simulink model "ofdm_txrx_supermimo_coded"  (Simulink 7.1)
settings: Solver=VariableStepDiscrete  SolverMode=SingleTasking  StartTime=0.0  StopTime=2*simOnly_simLength  RelTol=1e-3
PostLoadFcn:
  | ofdm_rx_supermimo_init
InitFcn:
  | ofdm_rx_supermimo_init
StartFcn:
  | ofdm_rx_supermimo_init
values from neighbouring files (symbols this model uses):
  Action0_Config = 0* 31*2^18 + 0*2^24 + PHY_AUTORESPONSE_REQ_GOODHDR + PHY_AUTORESPONSE_REQ_GOODPKT + PHY_AUTORESPONSE_REQ_FLAGA    [ofdm_tx_supermimo_init.m]
  Action1_Config = 0* 62*2^18 + PHY_AUTORESPONSE_REQ_GOODHDR + PHY_AUTORESPONSE_REQ_GOODPKT    [ofdm_tx_supermimo_init.m]
  Action2_Config = 0    [ofdm_tx_supermimo_init.m]
  Action3_Config = 0    [ofdm_tx_supermimo_init.m]
  Action4_Config = 0    [ofdm_tx_supermimo_init.m]
  Action5_Config = 0    [ofdm_tx_supermimo_init.m]
  ActionID_AFTransmit = 31    [ofdm_tx_supermimo_init.m]
  ActionID_Disabled = 0    [ofdm_tx_supermimo_init.m]
  ActionID_SetFlagA = 62    [ofdm_tx_supermimo_init.m]
  ActionID_SetFlagB = 61    [ofdm_tx_supermimo_init.m]
  BER_RAM_init_size = 2048    [ofdm_tx_supermimo_init.m]
  channel_h = [1 0; 1 0] * 0.3    [ofdm_tx_supermimo_init.m]
  CPLength = 16    [ofdm_tx_supermimo_init.m]
  FEC_Config = fec_coding_en*1 + fec_soft_dec*2 + fec_qpsk_scl*2^4 + fec_16qam_scl*2^8    (= 4195)    [ofdm_tx_supermimo_init.m]
  longCorr_coef_i = [-4*real(LTS) 0]    [ofdm_rx_supermimo_init.m]
  longCorr_coef_q = [4*imag(LTS) 0]    [ofdm_rx_supermimo_init.m]
  Match0_Config = 0    [ofdm_tx_supermimo_init.m]
  Match1_Config = 0    [ofdm_tx_supermimo_init.m]
  Match2_Config = 0    [ofdm_tx_supermimo_init.m]
  Match3_Config = 0    [ofdm_tx_supermimo_init.m]
  Match4_Config = 0    [ofdm_tx_supermimo_init.m]
  Match5_Config = 0    [ofdm_tx_supermimo_init.m]
  Match6_Config = 0    [ofdm_tx_supermimo_init.m]
  Match7_Config = 0    [ofdm_tx_supermimo_init.m]
  max_CP_length = 16    [ofdm_tx_supermimo_init.m]
  max_num_baseRateSymbols = 31    [ofdm_tx_supermimo_init.m]
  max_num_subcarriers = 64    [ofdm_tx_supermimo_init.m]
  max_num_trainingSymbols = 15    [ofdm_tx_supermimo_init.m]
  max_numBytes = 16384    [ofdm_tx_supermimo_init.m]
  max_OFDM_symbols = 1023    [ofdm_tx_supermimo_init.m]
  modConstellation_qam16 = .75*[1 3 -1 -3]./3    [ofdm_tx_supermimo_init.m]
  modConstellation_qam64 = 0.875*[3 1 5 7 -3 -1 -5 -7]./7    [ofdm_tx_supermimo_init.m]
  modConstellation_qpsk = [1 -1]./sqrt(2)    [ofdm_tx_supermimo_init.m]
  numBaseRateSymbols = numBaseRateSymbols *2    [ofdm_tx_supermimo_init.m]
  numSubcarriers = 64    [ofdm_tx_supermimo_init.m]
  numTrainingSymbols = 2    [ofdm_tx_supermimo_init.m]
  pilot_indicies = 7 + ( (64-21) * 2^8) + ( (64-7) * 2^16) + (21 * 2^24)    (= 356068103)    [ofdm_tx_supermimo_init.m]
  pilotValues = hex2dec('A57D') + (2^16 * hex2dec('5A82'))    [ofdm_tx_supermimo_init.m]
  pktByteNums = numHeaderBytes +  				byteIndex_numPayloadBytes(1)*2^8 +  				byteIndex_numPayloadBytes(2)*2^16 +  				byteIndex_simpleDynModMasks * 2^24    [ofdm_rx_supermimo_init.m]
  PktDet_Delay = 58+32 +      Symbol_Timing_Offset*2^7 +      0*2^16 +      (117* 2^24)    (= 1962935642)    [ofdm_rx_supermimo_init.m]
  pktTiming_count_payloadStart = pktTiming_count_lsStart + pktTiming_ls_num*pktTiming_ls_length    [ofdm_rx_supermimo_init.m]
  preamble = preamble_scale*[shortsyms_10 longsyms_2]    [ofdm_tx_supermimo_init.m]
  preamble_ROM = preamble_I_ROM + preamble_Q_ROM*2^16    [ofdm_tx_supermimo_init.m]
  RAM_init_size = max_numBytes    (= 16384)    [ofdm_tx_supermimo_init.m]
  reg_AF_Tx_Blanking = (320+80) + ( (320+80+79)*2^16)    (= 31392144)    [ofdm_rx_supermimo_init.m]
  reg_AF_Tx_Scaling = 2944    [ofdm_rx_supermimo_init.m]
  reg_Interrupt_PktBuf_Control = reg_InterruptControl +  	1 * 2^16 +  	0 * 2^24    (= 65536)    [ofdm_rx_supermimo_init.m]
  reg_PilotCalcParams = (4 * 2^16) + (minPilotChanMag)    (= 262184.96)    [ofdm_rx_supermimo_init.m]
  regRx_chanEst_minMag = ((0.5)*2^15) + ((0.5 * 2^15)*2^16)    (= 1073758208)    [ofdm_rx_supermimo_init.m]
  regRx_coarseCalcCorrection = round((1.3e-3 - 1.2982e-3)*2^32)    [ofdm_rx_supermimo_init.m]
  regRx_pilotCalcCorrection = round(1.00115 * 2^31)    [ofdm_rx_supermimo_init.m]
  regRx_preCFOoptions = 0 * 2^0 +  	1 * 2^1 +  	0    (= 2)    [ofdm_rx_supermimo_init.m]
  rx_controlBits = 1 * 2  		 + 1 * 4  		 + 0 * 8  		 + 1 * 16 * rx_SISO_Mode  		 + 1 * 32  		 + 1 * 64  		 + 1 * 128  		 + 1 * 256  		 + 1 * 512  		 + 1 * 1024  		 + 1 * 4096  		 + 0 * 16384  		 + 0 * 32768  		 + 1 * 65536  		 + 0 * 131072  		 + 0 * 262144  		 + 1 * 524288  		 + 1 * 1048576 * tx_Alamouti_Mode  		 + 0 * 2097152           + 1 * 33554432  		 + 0 * 4194304  		 + 0 * 8388608  		 + 0 * 16777216  		 + 1 *  ...(+49 chars)    [ofdm_rx_supermimo_init.m]
  Rx_PktDet_LongCorr_Params = LongCorrCounterLoad + 0*2^8 + 0*2^16 + 255*2^24    (= 4278190325)    [ofdm_rx_supermimo_init.m]
  Rx_PktDet_LongCorrThresholds = 8000    [ofdm_rx_supermimo_init.m]
  rx_postEq_scaling = round(rxScaling*2^11) * (1 + 2^16)    [ofdm_rx_supermimo_init.m]
  RxDataScrambling_Seq = [40 198 78 63 82 173 102 245 48 111 172 115 147 230 216 93 72 65 62 2 205 242 122 90 128 83 105 97 73 10 5 252]    [ofdm_tx_supermimo_init.m]
  RxReg_FixedPktLen = 0    [ofdm_rx_supermimo_init.m]
  rxReg_pktDetCorr = 2^00 * (floor(0.7*128)) +  	2^08 * (300) +  	0    [ofdm_rx_supermimo_init.m]
  shortSymbol_time = shortSymbol_time(1:16).'    [ofdm_tx_supermimo_init.m]
  simOnly_numSamples = length(preamble)+( (numSubcarriers+CPLength)*(numTrainingSymbols + numBaseRateSymbols + numFullRateSymbols/2) )    [ofdm_tx_supermimo_init.m]
  train = [train train]    [ofdm_tx_supermimo_init.m]
  tx_controlBits = (antB_preambleShift * 2^4) + (2^1 * tx_Alamouti_Mode) +  	(2^0 * tx_SISO_Mode) + 4*0 + 8*1 + 0*256 + 512*0 + 1024*0 +  	0*hex2dec('1000') + 0*hex2dec('2000') + 0*hex2dec('4000') +  	1*hex2dec('8000') + 1*hex2dec('10000') + hex2dec('20000') +  	0*hex2dec('100000')    [ofdm_tx_supermimo_init.m]
  tx_delays = (50*2^0) + (0*2^8) + (30*2^12)    (= 122930)    [ofdm_tx_supermimo_init.m]
  tx_scaling = (3072) + (12288 * 2^16)    (= 805309440)    [ofdm_tx_supermimo_init.m]
  TxDataScrambling_Seq = [40 198 78 63 82 173 102 245 48 111 172 115 147 230 216 93 72 65 62 2 205 242 122 90 128 83 105 97 73 10 5 252]    [ofdm_tx_supermimo_init.m]
  txReg_symbolCounts = (2^8 *numBaseRateSymbols ) + numTrainingSymbols    [ofdm_tx_supermimo_init.m]
  TxRx_FFTScaling = (3 * 2^0) +  	(2 * 2^2) +  	(1 * 2^4) +  	(1 * 2^6) +  	(1 * 2^8) +  	(0 * 2^10)    (= 347)    [ofdm_tx_supermimo_init.m]
undefined symbols (not defined in the file or in its folder): AntA, AntB, BaseRate, Down, gcbh, numBuffs, numBytes, Up, xlBlockLoadCallback, xlGetSimulinkPeriod, xlipgetpartsetting
libraries: xbsIndex_r4 x6000, simulink x4, dspsigops x4, commchan3 x2, dspsrcs4 x2, commrflib2 x2
(part 3 of 63)

== system ofdm_txrx_supermimo_coded/Memory-mapped Registers ==
  AutoReply Registers : Subsystem
  Rx Registers : Subsystem
  Static Parameters (might be registers someday) : Subsystem
  Tx Registers : Subsystem
  TxRx Registers : Subsystem

== system ofdm_txrx_supermimo_coded/OFDM Rx MIMO ==
  AntA_I : Inport  OutDataType=sfix(16)  OutScaling=2^0
  AntA_Q : Inport  Port=2  OutDataType=sfix(16)  OutScaling=2^0
  AntB_I : Inport  Port=3  OutDataType=sfix(16)  OutScaling=2^0
  AntB_Q : Inport  Port=4  OutDataType=sfix(16)  OutScaling=2^0
  ADC Inputs & Antenna Selection : Subsystem
  AF Buffers : Subsystem
  ChipScope : Subsystem
  Coarse Freq Correction : Subsystem
  Display : Display
  Display1 : Display
  Display2 : Display
  Divide : Product  Inputs=*/  OutDataType=sfix(16)  OutScaling=2^-10
  Equalizer & Packetizer : Subsystem
  FFT & Chan Est : Subsystem
  Long Corr HP : Subsystem
  Packet_Detection : Subsystem
  Packet_Timing_Control : Subsystem
  Rx Input : Scope  NumInputPorts=7
      settings: TimeRange=14600  YMin=-0.2~-0.2~-1~-1~0~0~0  YMax=0.2~0.2~1~1~1~22500~1  SaveName=ScopeData48
  Rx Reset Logic : Subsystem
  done{1..9} (9 alike) : xbsIndex_r4/Gateway Out  hdl_port=off  timing_constraint=None  locs_specified=off  LOCs={}  block_type=gatewayout
      settings: infoedit="Gateway out block.  Converts Xilinx fixed point inputs into ouputs of type Simulink integer, double, or fixed point.<P><P>Hardware notes:  In hardware these blocks become top level output ports or are discarded, depending on how they are configured."  xl_area=[0 0 0 0 1 0 0]  block_version=VER_STRING_GOES_HERE
  tag scope: Payload=global, PktDetected=global
  wires:
  Packet_Detection/1(Pkt Det) -> Packet_Timing_Control/1(Pkt Det), done1/1
  Packet_Timing_Control/1(Payload_Preamble) -> Equalizer & Packetizer/1(Payload), FFT & Chan Est/1(Payload), Packet_Detection/3(Payload), AF Buffers/2(Payload), [Payload], done7/1
  Long Corr HP/1(y) -> Packet_Timing_Control/2(Long CorrA), done2/1
  done1/1 -> Rx Input/5
  done4/1 -> Rx Input/1
  done5/1 -> Rx Input/2
  done3/1 -> Rx Input/3
  done6/1 -> Rx Input/4
  Coarse Freq Correction/2("AntA Q ") -> FFT & Chan Est/3(AntA Q), Long Corr HP/2(Q In)
  Coarse Freq Correction/3("AntB I ") -> FFT & Chan Est/4(AntB I)
  Coarse Freq Correction/4("AntB Q ") -> FFT & Chan Est/5(AntB Q)
  done2/1 -> Rx Input/6
  AntA_I/1 -> ADC Inputs & Antenna Selection/2("A I ")
  AntA_Q/1 -> ADC Inputs & Antenna Selection/3("A Q ")
  AntB_I/1 -> ADC Inputs & Antenna Selection/4("B I ")
  AntB_Q/1 -> ADC Inputs & Antenna Selection/5("B Q ")
  Equalizer & Packetizer/1(done) -> Packet_Timing_Control/3(Done), AF Buffers/1(PktDone)
  FFT & Chan Est/1(Y_I) -> Equalizer & Packetizer/2(Y_I)
  FFT & Chan Est/2(Y_Q) -> Equalizer & Packetizer/3(Y_Q)
  FFT & Chan Est/3(H-I) -> Equalizer & Packetizer/4(H-I)
  FFT & Chan Est/4(H-Q) -> Equalizer & Packetizer/5(H-Q)
  FFT & Chan Est/5(SubCar Index) -> Equalizer & Packetizer/6(SubCar Index)
  FFT & Chan Est/6(Sym Index) -> Equalizer & Packetizer/7(Sym Index)
  FFT & Chan Est/7(Vout) -> Equalizer & Packetizer/8(Vin)
  Packet_Timing_Control/2(PktReset) -> ADC Inputs & Antenna Selection/1(PktDetReset), Coarse Freq Correction/1(Reset), Packet_Detection/2(Reset)
  Packet_Timing_Control/3(PktDetected) -> [PktDetected], Coarse Freq Correction/2(PktDet), Packet_Detection/1(Pkt Active)
  Divide/1 -> Display/1
  [regRx_BER_Errors] -> done8/1
  [regRx_BER_TotalBits] -> done9/1
  ADC Inputs & Antenna Selection/1(A I) -> Coarse Freq Correction/3(AntA I), done4/1, AF Buffers/3(AntA_I)
  ADC Inputs & Antenna Selection/2(A Q) -> Coarse Freq Correction/4(AntA Q), done5/1, AF Buffers/4(AntA_Q)
  ADC Inputs & Antenna Selection/3(B I) -> Coarse Freq Correction/5(AntB I), done3/1
  ADC Inputs & Antenna Selection/4(B Q) -> Coarse Freq Correction/6(AntB Q), done6/1
  FFT & Chan Est/8(Phase I) -> Equalizer & Packetizer/9(Phases I)
  Coarse Freq Correction/1("AntA I ") -> FFT & Chan Est/2(AntA I), Long Corr HP/1(I In)
  done7/1 -> Rx Input/7
  FFT & Chan Est/9(Phase Q) -> Equalizer & Packetizer/10(Phases Q)
  done8/1 -> Divide/1, Display1/1
  done9/1 -> Divide/2, Display2/1

== system ofdm_txrx_supermimo_coded/OFDM Tx MIMO ==
  IFFT : Subsystem
  OutputBuffers : Subsystem
  OutputMuxes : Subsystem
  "Pre-Spin,  Filters & DACs" : Subsystem
  Training_Data : Subsystem  Orientation=left
  Tx Reset Logic : Subsystem
  TxControl : Subsystem
  TxStart : Subsystem
  AntA I : Outport  OutDataType=sfix(16)  OutScaling=2^0
  AntA Q : Outport  Port=2  OutDataType=sfix(16)  OutScaling=2^0
  AntB I : Outport  Port=3  OutDataType=sfix(16)  OutScaling=2^0
  AntB Q : Outport  Port=4  OutDataType=sfix(16)  OutScaling=2^0
  wires:
  TxStart/1(Start) -> TxControl/1(StartTx)
  Training_Data/1(tx_Q) -> IFFT/3(xn_Q)
  TxControl/7(Start IFFT) -> IFFT/4(Start)
  IFFT/3(xk_I) -> OutputBuffers/2(I)
  IFFT/4(xk_Q) -> OutputBuffers/3(Q)
  IFFT/1(xn_Index) -> Training_Data/7(xn_index)
  IFFT/5(xk_Index) -> OutputBuffers/4(Xk_index)
  OutputBuffers/1(AntA_I) -> OutputMuxes/2(AntA I)
  OutputBuffers/2(AntA_Q) -> OutputMuxes/3(AntA Q)
  TxControl/5(Training_Data) -> Training_Data/2(train_pay)
  TxControl/6(CPn_Data) -> Training_Data/1(CPn_Data)
  TxControl/4(BaseRate_En) -> Training_Data/3(BaseRate_En)
  OutputBuffers/5(Vout) -> OutputMuxes/6(vin)
  Training_Data/2(tx_I) -> IFFT/2(xn_I)
  OutputBuffers/6(Pkt Done) -> OutputMuxes/7(Pkt Done), Training_Data/6(packet_done), IFFT/5(Pkt Done), TxControl/2(PktDone)
  OutputBuffers/3(AntB_I) -> OutputMuxes/4(AntB I)
  OutputBuffers/4(AntB_Q) -> OutputMuxes/5(AntB Q)
  TxControl/1(Preamble Index) -> OutputMuxes/1(Pream Index)
  IFFT/2(Vout) -> OutputBuffers/1(IFFT Vout)
  TxControl/3(FFT En) -> Training_Data/4(FFT En)
  "Pre-Spin,  Filters & DACs"/1(" A_I") -> AntA I/1
  "Pre-Spin,  Filters & DACs"/2(" A_Q") -> AntA Q/1
  "Pre-Spin,  Filters & DACs"/3(" B_I") -> AntB I/1
  "Pre-Spin,  Filters & DACs"/4(" B_Q") -> AntB Q/1
  TxControl/2(Start) -> Training_Data/5(Start)
  Training_Data/3(Payload Done) -> IFFT/1(Payload Done)
  IFFT/6(LastSym) -> OutputBuffers/5(Last Sym)
  OutputMuxes/1(Payload) -> "Pre-Spin,  Filters & DACs"/1(Payload)
  OutputMuxes/2(" AntA I") -> "Pre-Spin,  Filters & DACs"/2(A_I)
  OutputMuxes/3(" AntA Q") -> "Pre-Spin,  Filters & DACs"/3(A_Q)
  OutputMuxes/4(" AntB I") -> "Pre-Spin,  Filters & DACs"/4(B_I)
  OutputMuxes/5(" AntB Q") -> "Pre-Spin,  Filters & DACs"/5(B_Q)

== system ofdm_txrx_supermimo_coded/Pkt Buffer BRAM Interface ==
  Addr Select : Subsystem
  BRAM_Addr : xbsIndex_r4/Gateway Out  hdl_port=on  timing_constraint=None  locs_specified=off  LOCs={}  block_type=gatewayout
      settings: infoedit="Gateway out block.  Converts Xilinx fixed point inputs into ouputs of type Simulink integer, double, or fixed point.<P><P>Hardware notes:  In hardware these blocks become top level output ports or are discarded, depending on how they are configured."  xl_area=[0, 0, 0, 0, 0, 0, 0]  block_version=VER_STRING_GOES_HERE
  BRAM_DataIn : xbsIndex_r4/Gateway In  arith_type=Unsigned  n_bits=64  bin_pt=0  quantization="Round  (unbiased: +/- Inf)"  overflow=Saturate  period=1  dbl_ovrd=off  timing_constraint=None  locs_specified=off  LOCs={}  block_type=gatewayin
      settings: infoedit="Gateway in block.  Converts inputs of type Simulink integer, double and fixed point to  Xilinx fixed point type.<P><P>Hardware notes:  In hardware these blocks become top level input ports."  block_version=VER_STRING_GOES_HERE
  BRAM_Dout : xbsIndex_r4/Gateway Out  hdl_port=on  timing_constraint=None  locs_specified=off  LOCs={}  block_type=gatewayout
      settings: infoedit="Gateway out block.  Converts Xilinx fixed point inputs into ouputs of type Simulink integer, double, or fixed point.<P><P>Hardware notes:  In hardware these blocks become top level output ports or are discarded, depending on how they are configured."  xl_area=[0, 0, 0, 0, 0, 0, 0]  block_version=VER_STRING_GOES_HERE
  BRAM_Reset : xbsIndex_r4/Gateway Out  hdl_port=on  timing_constraint=None  locs_specified=off  LOCs={}  block_type=gatewayout
      settings: infoedit="Gateway out block.  Converts Xilinx fixed point inputs into ouputs of type Simulink integer, double, or fixed point.<P><P>Hardware notes:  In hardware these blocks become top level output ports or are discarded, depending on how they are configured."  block_version=8.2.02
  BRAM_WEn : xbsIndex_r4/Gateway Out  hdl_port=on  timing_constraint=None  locs_specified=off  LOCs={}  block_type=gatewayout
      settings: infoedit="Gateway out block.  Converts Xilinx fixed point inputs into ouputs of type Simulink integer, double, or fixed point.<P><P>Hardware notes:  In hardware these blocks become top level output ports or are discarded, depending on how they are configured."  xl_area=[0, 0, 0, 0, 0, 0, 0]  block_version=VER_STRING_GOES_HERE
  Constant : xbsIndex_r4/Constant  arith_type=Unsigned  const=0  n_bits=1  bin_pt=0  explicit_period=on  period=1  equ=P=C  opselect=C  inp2=PCIN>>17  opr=+  inp1=P  carry=CIN  dbl_ovrd=off  block_type=constant
      settings: block_version=8.2.02
  Mux : xbsIndex_r4/Mux  inputs=2  en=off  latency=0  precision=Full  arith_type=Unsigned  n_bits=16  bin_pt=14  quantization=Truncate  overflow=Wrap  dbl_ovrd=off  block_type=mux
      settings: block_version=8.2.02
  PLB Pkt Buffer : Subsystem
  Pkt Buffer Addressing : Subsystem
  SIM ONLY! FlexBER Pkt Buffer : Subsystem
  tag scope: BRAM_Tx_DIn=global, Debug_BRAM_Addr=global
  wires:
  PLB Pkt Buffer/1(Data) -> BRAM_DataIn/1
  BRAM_DataIn/1 -> [BRAM_Tx_DIn]
  Constant/1 -> BRAM_Reset/1
  Mux/1 -> BRAM_Addr/1, [Debug_BRAM_Addr]
  BRAM_Dout/1 -> PLB Pkt Buffer/1(BRAM_Dout)
  BRAM_WEn/1 -> PLB Pkt Buffer/2(WEn)
  BRAM_Addr/1 -> PLB Pkt Buffer/3(Addr)
  Pkt Buffer Addressing/1(Tx_RdAddr) -> Mux/2
  Pkt Buffer Addressing/2(Rx_WrAddr) -> Mux/3
  Addr Select/1(Sel) -> Mux/1
  [BRAM_Rx_WrEn] -> BRAM_WEn/1
  [BRAM_Rx_DOut] -> BRAM_Dout/1

== system ofdm_txrx_supermimo_coded/Workspace Transmitter ==
  From Workspace : FromWorkspace  VariableName=rxAntI  SampleTime=4  Interpolate=off  OutputAfterFinalValue=Cyclic repetition
  From Workspace1 : FromWorkspace  VariableName=rxAntQ  SampleTime=4  Interpolate=off  OutputAfterFinalValue=Cyclic repetition
  RXreset : Constant  Value=0  OutDataType=sfix(16)  OutScaling=2^0  SampleTime=4
  A_I : Outport  OutDataType=sfix(16)  OutScaling=2^0
  A_Q : Outport  Port=2  OutDataType=sfix(16)  OutScaling=2^0
  B_I : Outport  Port=3  OutDataType=sfix(16)  OutScaling=2^0
  B_Q : Outport  Port=4  OutDataType=sfix(16)  OutScaling=2^0
  wires:
  From Workspace/1 -> A_I/1
  From Workspace1/1 -> A_Q/1
  RXreset/1 -> B_I/1, B_Q/1

== system ofdm_txrx_supermimo_coded/"Channel"/Delays ==
  In1 : Inport  OutDataType=sfix(16)  OutScaling=2^0
  In2 : Inport  Port=2  OutDataType=sfix(16)  OutScaling=2^0
  Integer Delay1 : simulink/Discrete/Integer Delay  vinit=0.0  samptime=-1  NumDelays=4
  Integer Delay2 : simulink/Discrete/Integer Delay  vinit=0.0  samptime=-1  NumDelays=4
  Out1 : Outport  OutDataType=sfix(16)  OutScaling=2^0
  Out2 : Outport  Port=2  OutDataType=sfix(16)  OutScaling=2^0
  wires:
  In1/1 -> Integer Delay1/1
  Integer Delay1/1 -> Out1/1
  Integer Delay2/1 -> Out2/1
  In2/1 -> Integer Delay2/1

== system ofdm_txrx_supermimo_coded/"Channel"/Freq Offset ==
  note: Optional additive carrier
      | Models effects of DC-offsets at Tx/Rx
      | Set gain=0.15 for realistic offset
  In1 : Inport  OutDataType=sfix(16)  OutScaling=2^0
  In2 : Inport  Port=2  OutDataType=sfix(16)  OutScaling=2^0
  Product4 : Product  OutDataType=sfix(16)  OutScaling=2^0  RndMeth=Zero
  Product5 : Product  OutDataType=sfix(16)  OutScaling=2^0  RndMeth=Zero
  Sine Wave : dspsrcs4/Sine Wave  Amplitude=1  Frequency=freqOffsetB/4  Phase=0  SampleMode=Discrete  OutComplex=Complex  CompMethod=Trigonometric fcn  TableSize=Speed  SampleTime=1  SamplesPerFrame=1  dataType=double  wordLen=16  udDataType=sfix(16)  fracBitsMode=Best precision  numFracBits=15  ResetState=Restart at time zero
  Sine Wave1 : dspsrcs4/Sine Wave  Amplitude=1  Frequency=freqOffsetA/4  Phase=0  SampleMode=Discrete  OutComplex=Complex  CompMethod=Trigonometric fcn  TableSize=Speed  SampleTime=1  SamplesPerFrame=1  dataType=double  wordLen=16  udDataType=sfix(16)  fracBitsMode=Best precision  numFracBits=15  ResetState=Restart at time zero
  Out1 : Outport  OutDataType=sfix(16)  OutScaling=2^0
  Out2 : Outport  Port=2  OutDataType=sfix(16)  OutScaling=2^0
  tag scope: freqOffset=global
  wires:
  Sine Wave/1 -> Product5/2
  In1/1 -> Product4/1
  In2/1 -> Product5/1
  Sine Wave1/1 -> Product4/2, [freqOffset]
  Product4/1 -> Out1/1
  Product5/1 -> Out2/1

== system ofdm_txrx_supermimo_coded/"Channel"/MIMO "Channel" ==
  In1 : Inport  OutDataType=sfix(16)  OutScaling=2^0
  In2 : Inport  Port=2  OutDataType=sfix(16)  OutScaling=2^0
  Add : Sum  OutDataType=sfix(16)  OutScaling=2^-10
  Add1 : Sum  OutDataType=sfix(16)  OutScaling=2^-10
  Constant : Constant  Value=h(1,1)  OutDataType=sfix(16)  OutScaling=2^0
  Constant1 : Constant  Value=h(2,1)  OutDataType=sfix(16)  OutScaling=2^0
  Constant2 : Constant  Value=h(1,2)  OutDataType=sfix(16)  OutScaling=2^0
  Constant3 : Constant  Value=h(2,2)  OutDataType=sfix(16)  OutScaling=2^0
  Display{,1..3} (4 alike) : Display
  Product{,1..3} (4 alike) : Product  OutDataType=sfix(16)  OutScaling=2^0  RndMeth=Zero
  Out1 : Outport  OutDataType=sfix(16)  OutScaling=2^0
  Out2 : Outport  Port=2  OutDataType=sfix(16)  OutScaling=2^0
  wires:
  Constant/1 -> Product/2, Display/1
  Product/1 -> Add/1
  In1/1 -> Product/1, Product2/1
  In2/1 -> Product1/2, Product3/2
  Constant1/1 -> Product1/1, Display1/1
  Product1/1 -> Add/2
  Constant2/1 -> Product2/2, Display2/1
  Product2/1 -> Add1/1
  Constant3/1 -> Product3/1, Display3/1
  Product3/1 -> Add1/2
  Add/1 -> Out1/1
  Add1/1 -> Out2/1

== system ofdm_txrx_supermimo_coded/Changelog/Through PHY 3.00i ==
  note: Export 2.01.r:
      | -Yet another pilot phase fix
  note: Export 2.01.s:
      | -Disabled the CFO correction filter (to save multipliers in the V4)
  note: Export 2.01.t:
      | -Changed SISO H_BB matrix value to 1 (from 0.5+0.5j)
  note: ========================================
      | Starting iterations for pre-spin/DF support
  note: Export 3.00.a:
      | -Started from 2.01.t (PHY for refdes14.1_fpgaV2_public)
      | -Re-generated memmap (sims are faster w/out EDK Processor block)
      | -Removed nearly all scopes (faster sims, less memory consumption)
      | -Removed match6/7 blocks and localHeader buffer in autoResponse system (we never used this,
      |   after figuring the flagA/flagB actions/conditions were enough)
      | -Removed CFO PI filter
      | -Moved phase normalization to immediately after pilot arctan
      | -Completely redesigned pilot insertion logic and phase estimation/tracking logic. Pilots in Alamouti mode
      |    are now interleaved in frequency, not time. Phase error corrections are applied to the current symbol,
      |    not the next one. Overall improvement in EVM should be good, especially in Alamouti mode.
      | -Fixed checksum bug in random payload mode (payloads were being flagged as all bad)
      | -Added readback registers for pilot-based and coarse CFO estimates
      | -Added readback register for whether A and B channels are used in pilot phase calculation (Alamouti mode only)
  note: Export 3.00.b:
      | -Added FFT overflow/AGC/pkt signals to ChipScope
  note: Export 3.00.c:
      | -Fixed pilot selection for single-path Alamouti
  note: Export 3.00.d:
      | -Added CFO pre-spin blocks
      |   -Multipliers in Tx path, before filters
      |   -Pilot-based CFO estimator
      |   -Per-packet-buffer CFO capture buffer
  note: Export 3.00.e:
      | -Changed pre-spin mults to conj-mults
  note: Export 3.00.f:
      | -Added pktDetection event counter
      | -Added logic to capture random Tx payloads to a pkt buffer
      | -Shifted autoTx delay up one bit (user's 8 bits are [8:1] of 9-bit delay)
      | -Fixed antB conj mult (was conjugating wrong argument)
  note: Export 3.00.g:
      | -Fixed autoTx delay comparison (to < instead of <=, so that Tx isn't always being asserted)
  note: Export 3.00.h:
      | -Fixed latency on pilotCFOest_en signals, bumped CFO capture buffer to T=1
      | -Fixed autoTx delay counter (now 9 bits, matching delay param)
      | -Updated LFSR polynomial
  note: Export 3.00.i:
      | -Redesigned pilot pre-CFO calculator, to use all received phases instead of trying to average just the last N.

== system ofdm_txrx_supermimo_coded/Changelog/Through PHY 3.00u ==
  note: Export 3.00.j:
      | -Fixed bug in pilot-CFO calc (added reset to unwrapping counter)
  note: Export 3.00.k:
      | -Fixed bug in random payload generation/recording (LFSR en toggled only once per pkt in 3.00j)
  note: Export 3.00.l:
      | -Fixed random payload mode (again) so capture & checksums will work.
  note: Export 3.00.m:
      | -Added precision and multiplication correction factor to pilotCFO estimator, based on experimental tests.
  note: Export 3.00.n:
      | -Added AutoTwoTx circuit, to optionally transmit every user-initiated packet twice with programmed delay
      | -Added external PktDetReset input to hold Rx PHY in reset via GPIO
  note: Export 3.00.o:
      | -Fixed timing of AutoTwoTx
      | -Added autoResponse action to force Tx antenna swap
      | -Added optional circuit to calculate/store channel magnitudes (and not raw I/Q)
  note: Export 3.00.p:
      | -Added hooks to disable random payload generation for autoResponse and autoTwoTx transmissions
      |    (don't want new payloads for slot2 when testing PHY stuff)
      | -Fixed pktBuf mixup on autoTwoTx transissions (shouldn't use autoResponse pktBuf assignment)
  note: Export 3.00.q:
      | -Rearranged coarse CFO calc (moved 1/2pi scaling just after arctan)
      | -Added coarseCFO correction adder
  note: Export 3.00.r:
      | -Added coarseCFO correction register to EDK processor block (was omitted in q)
  note: Export 3.00.t:
      | -Redesigned checksum subsystems; header now has real CRC-16, full packet has real CRC-32
  note: Export 3.00.s:
      | -Re-export of 3.00r with logic multiliers for V4 project
  note: Export 3.00.u:
      | -Added EVM buffers
      |   -One summing all EVM for a given subcarrier across symbols
      |   -One summing EVM across all subcarriers per symbol
      | -Changed antB preamble shift to add (delay relative to antA)
      | -Changed autoResponder delay to 9 bits {user8, 0}, so each user bit is a sample delay
      | -Renamed coarseCFO correction register

== system ofdm_txrx_supermimo_coded/Changelog/Through PHY 3.01f ==
  note: Export 3.00.v:
      | -Fixed EVM-per-sc logic to reset at start of each Rx packet
  note: Export 3.00.w:
      | -Fixed autoResponder timing (new CRC blocks were 2 cycles slower declaring good/bad payload)
  note: Export 3.00.x:
      | -Added extra delay bits for autoTx and reTx counters
      | -Increased int. bits for per-SC EVM accumulation
      | -Added external pktDet port
      | -Rearragned TxControlBits register bits
  note: Export 3.00.y:
      | -Added additional TxRunning outputs with programmable masks/delays (for faking pktDetecton)
      | -Rearranged TxControlBits register and added TxDelays register
  note: Export 3.00.z:
      | -Added logic to allow coarse CFO to be triggered only by external pktDet (so long correlation can be bypassed entirely)
      | -Added channel estimate magnitude checking to optionally ignore HAA or HBA when they're too small
      | -Added logic to optionally mask part of the AF transmission (to blank the now-noisy unused training symbol)
      | -Changed AF scaling so all AF samples are scaled by TxPayload scaling (only re-generated preamble gets scaled
      |     by TxPreamble scaling)
  note: Export 3.01.a:
      | -Fixed timing bug that blocked autoResponder flags
  note: Export 3.01.b:
      | -Added selectable filters for TxA I/Q
  note: Export 3.01.c:
      | -Added precision to long correlator
  note: Export 3.01.d:
      | -Added registers to mask input I/Q until AGC is done
  note: Export 3.01.e:
      | -Added extra longCorr threshold check
  note: Export 3.01.f:
      | -Removed dualThreshold logic (didn't help)
      | -Rebuilt preamble generator for realy cyclic shift of STS/LTS for antB (removed masking too)
      | -Added regs to fix length of Rx pkt (for testing "true" AF)
      | -Removed unused RxControlBits (reqShortCorr, dynPktLenEn)
      | -Added windowing for LongCorr
      | -Added optional conjugation of antB preamble
      | -Added conjugate-correlation output from LongCorr

== system ofdm_txrx_supermimo_coded/Changelog/Through refdes14.0 ==
  note: Export 2.00.x:
      | -Added register to capture autoTx delay (required for actions which require a Flag)
      | -Added better TxStart logic (posedge on TxStart gateway; OR'd AF_TxDone for TxRunning SR reset)
      | -Screwed with precision in equalizer (more fractional bits, knowing abs(H)<1)
      | -Register phase error to chipscope/debug port
      | -Fixed TxPktLength%12==0 problem
      | -Adjust pktDet RSSI for RF gain change
      | -Added muxes to bypass Tx interp filters
  note: Export 2.00.y:
      | -Added option to use diff(phaseError) for fine CFO
      | -Shortened coarse CFO window to 68 samles (from 72)
      | -CFO delay should be > 0 (already settable in software)
      | -Increased precision of input to phase error scaling/lookup (remember to update constant in software!)
  note: Export 2.00.z:
      | -Shortened coarse CFO window to 64 samples (from 68)
  note: Export 2.00.z:
      | -Shortened coarse CFO window to 64 samples (from 68)
  note: Export 2.01.a:
      | -Added sanity checking for max phase diff in coarse CFO calc
      | -Added force reset of coarse CFO output when pkt not detected (coarse CFO was non-zero inter-packets before?)
      | -Added preCFO at Tx
      | -Added Sw reset of autoResponse flagA/B (via RxControlBits register)
      | -Modified action registers to include usePreCFO bit
      | -Increased accuracy/latency of coarse CFO arctan (11 cycles to 15)
      | -Reworked coarse CFO control (running sum, uses both longCorr crossings)
      | -Inverted training symbol; better PAPR in time domain this way (don't know why it was negated before)
  note: Export 2.01.b:
      | -Fixed action register connections (only action0 was connected in 2.01a)
  note: Export 2.01.c:
      | -Fixed potential stale state bits in the Tx control; hopefully the cause of the transmit-forever-on-reset condition we see occasionally
  note: Export 2.01.d:
      | -Added condition to capturing preCFO phaseInc- if either autoResponse flag is setup, it won't update the preCFO value
  note: Export 2.01.e:
      | -Added software control of pos/neg/reg for preCFO phaseInc
      | -Disabled payload scaling for AF Tx (preamble * afScaling for AF waveform now)
      | -BUG: DDS I/Q were connected at Tx for all Tx - maybe not a problem?
  note: Export 2.01.f:
      | -Fixed DDS I/Q bug
      | -Fixed support for non-QPSK baseRate in Alamouti mode
      | -Added TxReg bit to enable preCFO for all Tx pkts
      | -Added DDS_I to chipscope
      | -Disabled generation of chan est buf (to save BRAM for now)
  note: Export 2.01.g:
      | -Fixed EVM for BPSK
      | -Added option to average all 8 pilot tones (instead of 2x average of 4)
      | -Fixed phase tracking latency bug
  note: Export 2.01.h:
      | -Added precision to PN tracking 1/symNumber lookup table
      | -Added new packet-long CFO estimator
      | -Delayed TxDone by 8 samples
      | -Moved Tx scaling post-interp-filters
      | -Tx PreCFO mult is now pre-scaling, to avoid overflow
  note: Export 2.01.i:
      | -Removed packet-long CFO estimator
      | -Removed preCFO logic
      | -Added post-interp scaling for all four DAC outputs
      | -Un-disabled channel estimate shared mem
      | -Removed DDS from ChipScope
      | -Disabled AF buffers (still a work in progress)
      | -Adjusted timing of preamble/payload switch
  note: Export 2.01.j:
      | -Attempted re-design of phase tracking
  note: Export 2.01.k:
      | -Second attempt at redesigning phase tracking system. Phase
      | errors can now exceed 2*pi, to track larger residual CFO during
      | longer packets.
  note: Export 2.01.l:
      | -Fixed timing of phase correction block for Alamouti Rx
      | -Fixed (very) long-standing bug with 64-QAM checksums (false errors for some pkt lengths)
  note: Export 2.01.m:
      | -Fixed Tx control symbol counter (caused error for long Alamouti pkts)
  note: Export 2.01.n:
      | -Un-disabled AF buffer subsystem
  note: Export 2.01.o:
      | -Added logic to cap maximum pilot phase difference (otherwise a single noisy pilot can
      |    corrupt an entire packet by screwing up the phase tracking system)
  note: Export 2.01.p:
      | -Rebuilt pilot phase difference calc (previous "fix" made things worse...)
  note: Export 2.01.q:
      | -Yet another pilot phase fix
